simulink library "Renault_Library"  (Simulink 8.2)
settings: Solver=ode3  StartTime=0.0  StopTime=10.0  FixedStep=0.020  RelTol=1e-3
undefined symbols (not defined in the file or in its folder): ABC, AssystemProposal, b, chkval, CoeffA_C, CoeffB_C, CoeffMean_C, ConfTime_C, DiagDelay_C, DividePercent, FailureConfActive_C, FallingEdgeDuration_C, HighLevelThresholdVoltage_C, HighLevelVoltage_C, HighLevelVoltageTunable_C, InitValue_C, InputVoltRangeMax_C, InputVoltRangeMin_C, LowActiveContact_C, LowLevelThresholdVoltage_C, LowLevelVoltage_C, MaxOperatingVoltage_C, MinOperatingVoltage_C, mV2V_conversion, OUT1, OutOfRangeHigh_C, OutOfRangeLow_C, PinSupplySaturationMax, PinSupplySaturationMin, Res1Value_C, Res2Value_C, Res3Value_C, RisingEdgeDuration_C, RSA_CANSignalFailMonitoring, SampleTime_C, service14Table, service19Table, service22Table, service24Table, service28Table, service2ETable, service2FTable, service31Table, service87Table, SizeXStateDID2FTable, SizeXTable14, SizeXTable19, SizeXTable22, SizeXTable24, SizeXTable28, SizeXTable2E, SizeXTable2F, SizeXTable31, SizeXTable87, SizeYStateDID2FTable, SizeYTable14, SizeYTable19, SizeYTable22, SizeYTable24, SizeYTable28 ... (+22)
undefined symbols (not defined in the file): BatteryVoltage_ActivationThreshold_C, FrameStatus_AbsentValue_C, FrameStatus_ConfAbsentValue_C, MemThreshold_C, NAMEOFTHEMODEL_SAMPLE_TIME_CST, NbAbs1_C, NbConfAbs1_C, NbConfPres1_C, NbPres1_C, NOMDUSIGNAL_FaultConfTime_C, NOMDUSIGNAL_FaultRehabTime_C, NOMDUSIGNAL_switchvalue_C, NOMDUSIGNAL_unavailablevalue_C, PeriodFrame1_C, ResetTime_C, WakeUpTime_C
libraries: Renault_Library x112, simulink x75
(part 18 of 32)

== system Renault_Library/Services ECU/Diag/RSA_DiagnosticManagement/DIAGNOSTIC_SANCTION_TOP/Service11/SubFunction_In_Active_Session_Service11 ==
  DIAG_BUFFER_REQUEST : Inport
  SESSION : Inport  Port=2
  BitwiseOperator1 : simulink/Logic and Bit Operations/Bitwise Operator  logicop=AND  UseBitMask=on  BitMask=bin2dec('01111111')  BitMaskRealWorld=Stored Integer
  Constant{,11,13,15,18..20,22,24,27..28,35,37,39,42..43,49,51,53,56} (20 alike) : Constant  Value=true  OutDataTypeStr=boolean  SampleTime=-1
  Constant1 : Constant  Value=TYP_SESSION_ACTIVE.PROGRAMMING_SESSION  OutDataTypeStr=Inherit: Inherit via back propagation  SampleTime=-1
  Constant10 : Constant  Value=EnableRapidPowerShutDownSession1  OutDataTypeStr=Inherit: Inherit via back propagation  SampleTime=-1
  Constant12 : Constant  Value=HardResetSession1  OutDataTypeStr=Inherit: Inherit via back propagation  SampleTime=-1
  Constant14 : Constant  Value=KeyOffResetSession1  OutDataTypeStr=Inherit: Inherit via back propagation  SampleTime=-1
  Constant16 : Constant  Value=SoftResetSession1  OutDataTypeStr=Inherit: Inherit via back propagation  SampleTime=-1
  Constant17 : Constant  Value=DisableRapidPowerShutDownSession1  OutDataTypeStr=Inherit: Inherit via back propagation  SampleTime=-1
  Constant2 : Constant  Value=TYP_SESSION_ACTIVE.EXTENDED_SESSION  OutDataTypeStr=Inherit: Inherit via back propagation  SampleTime=-1
  Constant21 : Constant  Value=HardResetSession2  OutDataTypeStr=Inherit: Inherit via back propagation  SampleTime=-1
  Constant23 : Constant  Value=KeyOffResetSession2  OutDataTypeStr=Inherit: Inherit via back propagation  SampleTime=-1
  Constant25 : Constant  Value=SoftResetSession2  OutDataTypeStr=Inherit: Inherit via back propagation  SampleTime=-1
  Constant26 : Constant  Value=DisableRapidPowerShutDownSession2  OutDataTypeStr=Inherit: Inherit via back propagation  SampleTime=-1
  Constant{4,29,44,58} (4 alike) : Constant  Value=4  OutDataTypeStr=Inherit: Inherit via back propagation  SampleTime=-1
  Constant3 : Constant  Value=TYP_SESSION_ACTIVE.SAFETY_SYSTEM_SESSION  OutDataTypeStr=Inherit: Inherit via back propagation  SampleTime=-1
  Constant{5,30,45,59} (4 alike) : Constant  Value=5  OutDataTypeStr=Inherit: Inherit via back propagation  SampleTime=-1
  Constant{6,31,46,60} (4 alike) : Constant  Value=3  OutDataTypeStr=Inherit: Inherit via back propagation  SampleTime=-1
  Constant{32,47,61,65} (4 alike) : Constant  Value=2  OutDataTypeStr=Inherit: Inherit via back propagation  SampleTime=-1
  Constant{7,33,48,62} (4 alike) : Constant  OutDataTypeStr=Inherit: Inherit via back propagation  SampleTime=-1
  Constant34 : Constant  Value=EnableRapidPowerShutDownSession2  OutDataTypeStr=Inherit: Inherit via back propagation  SampleTime=-1
  Constant36 : Constant  Value=HardResetSession3  OutDataTypeStr=Inherit: Inherit via back propagation  SampleTime=-1
  Constant38 : Constant  Value=KeyOffResetSession3  OutDataTypeStr=Inherit: Inherit via back propagation  SampleTime=-1
  Constant40 : Constant  Value=SoftResetSession3  OutDataTypeStr=Inherit: Inherit via back propagation  SampleTime=-1
  Constant41 : Constant  Value=DisableRapidPowerShutDownSession3  OutDataTypeStr=Inherit: Inherit via back propagation  SampleTime=-1
  Constant50 : Constant  Value=HardResetSession4  OutDataTypeStr=Inherit: Inherit via back propagation  SampleTime=-1
  Constant52 : Constant  Value=KeyOffResetSession4  OutDataTypeStr=Inherit: Inherit via back propagation  SampleTime=-1
  Constant54 : Constant  Value=SoftResetSession4  OutDataTypeStr=Inherit: Inherit via back propagation  SampleTime=-1
  Constant55 : Constant  Value=DisableRapidPowerShutDownSession4  OutDataTypeStr=Inherit: Inherit via back propagation  SampleTime=-1
  Constant63 : Constant  Value=EnableRapidPowerShutDownSession4  OutDataTypeStr=Inherit: Inherit via back propagation  SampleTime=-1
  Constant8 : Constant  Value=TYP_SESSION_ACTIVE.DEFAULT_SESSION  OutDataTypeStr=Inherit: Inherit via back propagation  SampleTime=-1
  Constant9 : Constant  Value=EnableRapidPowerShutDownSession3  OutDataTypeStr=Inherit: Inherit via back propagation  SampleTime=-1
  LogicalOperator{,1..3,5..9,11..15,17..21,23,25..44} (40 alike) : Logic  OutDataTypeStr=boolean
  LogicalOperator{4,10,16,22} (4 alike) : Logic  Operator=OR  Inputs=5  OutDataTypeStr=boolean
  LogicalOperator24 : Logic  Operator=OR  Inputs=4  OutDataTypeStr=boolean
  RelationalOperator{1..43,45} (44 alike) : RelationalOperator  Operator===  OutDataTypeStr=boolean  InputSameDT=on
  Selector : Selector  InputPortWidth=4095  IndexOptions=Index vector (dialog)  Indices=[2]  OutputSizes=1
  SUBFUNCTION_SUPPORTED_IN_ACTIVE_SESSION : Outport
  wires:
  [Number_Subfunction] -> RelationalOperator40/1
  [Number_Subfunction] -> RelationalOperator38/1
  [Number_Subfunction] -> RelationalOperator41/1
  [Number_Subfunction] -> RelationalOperator39/1
  [Number_Subfunction] -> RelationalOperator42/1
  [Number_Subfunction] -> RelationalOperator31/1
  [Number_Subfunction] -> RelationalOperator29/1
  [Number_Subfunction] -> RelationalOperator32/1
  [Number_Subfunction] -> RelationalOperator30/1
  [Number_Subfunction] -> RelationalOperator33/1
  [Number_Subfunction] -> RelationalOperator21/1
  [Number_Subfunction] -> RelationalOperator19/1
  [Number_Subfunction] -> RelationalOperator22/1
  [Number_Subfunction] -> RelationalOperator20/1
  [Number_Subfunction] -> RelationalOperator23/1
  [Number_Subfunction] -> RelationalOperator5/1
  [Number_Subfunction] -> RelationalOperator4/1
  [Number_Subfunction] -> RelationalOperator6/1
  [Number_Subfunction] -> RelationalOperator45/1
  [Number_Subfunction] -> RelationalOperator7/1
  BitwiseOperator1/1 -> [Number_Subfunction]
  LogicalOperator24/1 -> SUBFUNCTION_SUPPORTED_IN_ACTIVE_SESSION/1
  LogicalOperator4/1 -> LogicalOperator24/1
  LogicalOperator22/1 -> LogicalOperator24/4
  LogicalOperator16/1 -> LogicalOperator24/3
  LogicalOperator10/1 -> LogicalOperator24/2
  RelationalOperator22/1 -> LogicalOperator32/1
  RelationalOperator19/1 -> LogicalOperator33/1
  RelationalOperator23/1 -> LogicalOperator30/1
  LogicalOperator32/1 -> LogicalOperator8/2
  Constant31/1 -> RelationalOperator22/2
  LogicalOperator33/1 -> LogicalOperator9/2
  LogicalOperator34/1 -> LogicalOperator11/2
  RelationalOperator21/1 -> LogicalOperator34/1
  Constant30/1 -> RelationalOperator21/2
  Constant29/1 -> RelationalOperator19/2
  RelationalOperator20/1 -> LogicalOperator31/1
  Constant32/1 -> RelationalOperator20/2
  RelationalOperator1/1 -> LogicalOperator30/2, LogicalOperator31/2, LogicalOperator32/2, LogicalOperator33/2, LogicalOperator34/2
  LogicalOperator30/1 -> LogicalOperator5/2
  LogicalOperator31/1 -> LogicalOperator7/2
  LogicalOperator11/1 -> LogicalOperator10/5
  RelationalOperator17/1 -> LogicalOperator11/1
  Constant27/1 -> RelationalOperator17/2
  Constant26/1 -> RelationalOperator17/1
  LogicalOperator9/1 -> LogicalOperator10/4
  LogicalOperator5/1 -> LogicalOperator10/1
  LogicalOperator8/1 -> LogicalOperator10/3
  LogicalOperator7/1 -> LogicalOperator10/2
  RelationalOperator18/1 -> LogicalOperator9/1
  Constant24/1 -> RelationalOperator18/2
  Constant34/1 -> RelationalOperator18/1
  RelationalOperator16/1 -> LogicalOperator8/1
  Constant25/1 -> RelationalOperator16/1
  Constant22/1 -> RelationalOperator16/2
  RelationalOperator15/1 -> LogicalOperator7/1
  Constant23/1 -> RelationalOperator15/1
  Constant20/1 -> RelationalOperator15/2
  RelationalOperator24/1 -> LogicalOperator5/1
  Constant19/1 -> RelationalOperator24/2
  Constant21/1 -> RelationalOperator24/1
  Constant33/1 -> RelationalOperator23/2
  RelationalOperator41/1 -> LogicalOperator42/1
  RelationalOperator38/1 -> LogicalOperator43/1
  RelationalOperator42/1 -> LogicalOperator40/1
  LogicalOperator42/1 -> LogicalOperator20/2
  Constant60/1 -> RelationalOperator41/2
  LogicalOperator43/1 -> LogicalOperator21/2
  LogicalOperator44/1 -> LogicalOperator23/2
  RelationalOperator40/1 -> LogicalOperator44/1
  Constant59/1 -> RelationalOperator40/2
  Constant58/1 -> RelationalOperator38/2
  RelationalOperator39/1 -> LogicalOperator41/1
  Constant61/1 -> RelationalOperator39/2
  RelationalOperator3/1 -> LogicalOperator40/2, LogicalOperator41/2, LogicalOperator42/2, LogicalOperator43/2, LogicalOperator44/2
  LogicalOperator40/1 -> LogicalOperator18/2
  LogicalOperator41/1 -> LogicalOperator19/2
  LogicalOperator23/1 -> LogicalOperator22/5
  RelationalOperator36/1 -> LogicalOperator23/1
  Constant56/1 -> RelationalOperator36/2
  Constant55/1 -> RelationalOperator36/1
  LogicalOperator21/1 -> LogicalOperator22/4
  LogicalOperator18/1 -> LogicalOperator22/1
  LogicalOperator20/1 -> LogicalOperator22/3
  LogicalOperator19/1 -> LogicalOperator22/2
  RelationalOperator37/1 -> LogicalOperator21/1
  Constant53/1 -> RelationalOperator37/2
  Constant63/1 -> RelationalOperator37/1
  RelationalOperator35/1 -> LogicalOperator20/1
  Constant54/1 -> RelationalOperator35/1
  Constant51/1 -> RelationalOperator35/2
  RelationalOperator34/1 -> LogicalOperator19/1
  Constant52/1 -> RelationalOperator34/1
  Constant49/1 -> RelationalOperator34/2
  RelationalOperator43/1 -> LogicalOperator18/1
  Constant43/1 -> RelationalOperator43/2
  Constant50/1 -> RelationalOperator43/1
  Constant62/1 -> RelationalOperator42/2
  RelationalOperator32/1 -> LogicalOperator37/1
  RelationalOperator29/1 -> LogicalOperator38/1
  RelationalOperator33/1 -> LogicalOperator35/1
  LogicalOperator37/1 -> LogicalOperator14/2
  Constant46/1 -> RelationalOperator32/2
  LogicalOperator38/1 -> LogicalOperator15/2
  LogicalOperator39/1 -> LogicalOperator17/2
  RelationalOperator31/1 -> LogicalOperator39/1
  Constant45/1 -> RelationalOperator31/2
  Constant44/1 -> RelationalOperator29/2
  RelationalOperator30/1 -> LogicalOperator36/1
  Constant47/1 -> RelationalOperator30/2
  RelationalOperator2/1 -> LogicalOperator35/2, LogicalOperator36/2, LogicalOperator37/2, LogicalOperator38/2, LogicalOperator39/2
  LogicalOperator35/1 -> LogicalOperator12/2
  LogicalOperator36/1 -> LogicalOperator13/2
  LogicalOperator17/1 -> LogicalOperator16/5
  RelationalOperator27/1 -> LogicalOperator17/1
  Constant42/1 -> RelationalOperator27/2
  Constant41/1 -> RelationalOperator27/1
  LogicalOperator15/1 -> LogicalOperator16/4
  LogicalOperator12/1 -> LogicalOperator16/1
  LogicalOperator14/1 -> LogicalOperator16/3
  LogicalOperator13/1 -> LogicalOperator16/2
  RelationalOperator28/1 -> LogicalOperator15/1
  Constant39/1 -> RelationalOperator28/2
  Constant9/1 -> RelationalOperator28/1
  RelationalOperator26/1 -> LogicalOperator14/1
  Constant40/1 -> RelationalOperator26/1
  Constant37/1 -> RelationalOperator26/2
  RelationalOperator25/1 -> LogicalOperator13/1
  Constant38/1 -> RelationalOperator25/1
  Constant35/1 -> RelationalOperator25/2
  RelationalOperator8/1 -> LogicalOperator12/1
  Constant28/1 -> RelationalOperator8/2
  Constant36/1 -> RelationalOperator8/1
  Constant48/1 -> RelationalOperator33/2
  RelationalOperator6/1 -> LogicalOperator27/1
  RelationalOperator4/1 -> LogicalOperator28/1
  RelationalOperator7/1 -> LogicalOperator25/1
  LogicalOperator27/1 -> LogicalOperator2/2
  Constant6/1 -> RelationalOperator6/2
  LogicalOperator28/1 -> LogicalOperator3/2
  LogicalOperator29/1 -> LogicalOperator6/2
  RelationalOperator5/1 -> LogicalOperator29/1
  Constant5/1 -> RelationalOperator5/2
  Constant4/1 -> RelationalOperator4/2
  RelationalOperator45/1 -> LogicalOperator26/1
  Constant65/1 -> RelationalOperator45/2
  RelationalOperator9/1 -> LogicalOperator25/2, LogicalOperator26/2, LogicalOperator27/2, LogicalOperator28/2, LogicalOperator29/2
  LogicalOperator25/1 -> LogicalOperator/2
  LogicalOperator26/1 -> LogicalOperator1/2
  LogicalOperator6/1 -> LogicalOperator4/5
  RelationalOperator13/1 -> LogicalOperator6/1
  Constant18/1 -> RelationalOperator13/2
  Constant17/1 -> RelationalOperator13/1
  LogicalOperator3/1 -> LogicalOperator4/4
  LogicalOperator/1 -> LogicalOperator4/1
  LogicalOperator2/1 -> LogicalOperator4/3
  LogicalOperator1/1 -> LogicalOperator4/2
  RelationalOperator14/1 -> LogicalOperator3/1
  Constant15/1 -> RelationalOperator14/2
  Constant10/1 -> RelationalOperator14/1
  RelationalOperator12/1 -> LogicalOperator2/1
  Constant16/1 -> RelationalOperator12/1
  Constant13/1 -> RelationalOperator12/2
  RelationalOperator11/1 -> LogicalOperator1/1
  Constant14/1 -> RelationalOperator11/1
  Constant11/1 -> RelationalOperator11/2
  RelationalOperator10/1 -> LogicalOperator/1
  Constant/1 -> RelationalOperator10/2
  Constant12/1 -> RelationalOperator10/1
  Constant7/1 -> RelationalOperator7/2
  DIAG_BUFFER_REQUEST/1 -> Selector/1
  Selector/1 -> BitwiseOperator1/1
  Constant3/1 -> RelationalOperator3/2
  Constant2/1 -> RelationalOperator2/2
  Constant1/1 -> RelationalOperator1/2
  SESSION/1 -> RelationalOperator3/1, RelationalOperator2/1, RelationalOperator1/1, RelationalOperator9/1
  Constant8/1 -> RelationalOperator9/2

== system Renault_Library/Services ECU/Diag/RSA_DiagnosticManagement/DIAGNOSTIC_SANCTION_TOP/Service11/SubFunction_Supported_Service11 ==
  DIAG_BUFFER_REQUEST : Inport
  BitwiseOperator1 : simulink/Logic and Bit Operations/Bitwise Operator  logicop=AND  UseBitMask=on  BitMask=bin2dec('01111111')  BitMaskRealWorld=Stored Integer
  BitwiseOperator4 : simulink/Logic and Bit Operations/Bitwise Operator  logicop=AND  UseBitMask=on  BitMask=bin2dec('10000000')  BitMaskRealWorld=Stored Integer
  Constant : Constant  OutDataTypeStr=Inherit: Inherit via back propagation  SampleTime=-1
  Constant1 : Constant  Value=2  OutDataTypeStr=Inherit: Inherit via back propagation  SampleTime=-1
  Constant10 : Constant  Value=EnableRapidPowerShutDown  OutDataTypeStr=Inherit: Inherit via back propagation  SampleTime=-1
  Constant{5,7,9,11..12} (5 alike) : Constant  Value=true  OutDataTypeStr=boolean  SampleTime=-1
  Constant16 : Constant  OutDataTypeStr=Inherit: Inherit via back propagation  SampleTime=-1
  Constant17 : Constant  Value=DisableRapidPowerShutDown  OutDataTypeStr=Inherit: Inherit via back propagation  SampleTime=-1
  Constant19 : Constant  Value=5  OutDataTypeStr=Inherit: Inherit via back propagation  SampleTime=-1
  Constant2 : Constant  Value=3  OutDataTypeStr=Inherit: Inherit via back propagation  SampleTime=-1
  Constant3 : Constant  Value=HardReset  OutDataTypeStr=Inherit: Inherit via back propagation  SampleTime=-1
  Constant4 : Constant  Value=4  OutDataTypeStr=Inherit: Inherit via back propagation  SampleTime=-1
  Constant6 : Constant  Value=KeyOffReset  OutDataTypeStr=Inherit: Inherit via back propagation  SampleTime=-1
  Constant8 : Constant  Value=SoftReset  OutDataTypeStr=Inherit: Inherit via back propagation  SampleTime=-1
  LogicalOperator{,1..3,6} (5 alike) : Logic  OutDataTypeStr=boolean
  LogicalOperator4 : Logic  Operator=OR  Inputs=5  OutDataTypeStr=boolean
  RelationalOperator{,1..7,12..13,16} (11 alike) : RelationalOperator  Operator===  OutDataTypeStr=boolean  InputSameDT=on
  Selector : Selector  InputPortWidth=4095  IndexOptions=Index vector (dialog)  Indices=[2]  OutputSizes=1
  ShiftArithmetic3 : ArithShift  BitShiftNumber=7
  SUPPRESS_POS_RES : Outport
  SUBFUNCTION_SUPPORTED : Outport  Port=2
  wires:
  LogicalOperator6/1 -> LogicalOperator4/5
  RelationalOperator12/1 -> LogicalOperator6/2
  RelationalOperator13/1 -> LogicalOperator6/1
  Constant12/1 -> RelationalOperator13/2
  Constant17/1 -> RelationalOperator13/1
  Constant19/1 -> RelationalOperator12/2
  BitwiseOperator1/1 -> RelationalOperator12/1, RelationalOperator3/1, RelationalOperator2/1, RelationalOperator1/1, RelationalOperator/1
  Constant16/1 -> RelationalOperator16/2
  Selector/1 -> BitwiseOperator1/1, BitwiseOperator4/1
  ShiftArithmetic3/1 -> RelationalOperator16/1
  BitwiseOperator4/1 -> ShiftArithmetic3/1
  RelationalOperator16/1 -> SUPPRESS_POS_RES/1
  RelationalOperator3/1 -> LogicalOperator3/2
  LogicalOperator4/1 -> SUBFUNCTION_SUPPORTED/1
  LogicalOperator3/1 -> LogicalOperator4/4
  LogicalOperator/1 -> LogicalOperator4/1
  LogicalOperator2/1 -> LogicalOperator4/3
  LogicalOperator1/1 -> LogicalOperator4/2
  RelationalOperator7/1 -> LogicalOperator3/1
  Constant11/1 -> RelationalOperator7/2
  Constant10/1 -> RelationalOperator7/1
  RelationalOperator6/1 -> LogicalOperator2/1
  RelationalOperator2/1 -> LogicalOperator2/2
  Constant8/1 -> RelationalOperator6/1
  Constant9/1 -> RelationalOperator6/2
  RelationalOperator5/1 -> LogicalOperator1/1
  RelationalOperator1/1 -> LogicalOperator1/2
  Constant6/1 -> RelationalOperator5/1
  Constant7/1 -> RelationalOperator5/2
  RelationalOperator4/1 -> LogicalOperator/1
  RelationalOperator/1 -> LogicalOperator/2
  Constant5/1 -> RelationalOperator4/2
  Constant3/1 -> RelationalOperator4/1
  Constant4/1 -> RelationalOperator3/2
  Constant2/1 -> RelationalOperator2/2
  Constant1/1 -> RelationalOperator1/2
  Constant/1 -> RelationalOperator/2
  DIAG_BUFFER_REQUEST/1 -> Selector/1

== system Renault_Library/Services ECU/Diag/RSA_DiagnosticManagement/DIAGNOSTIC_SANCTION_TOP/Service14/Check_Lenght_Message_Service14 ==
  REQ_DATA_NUMBER : Inport
  Constant1 : Constant  Value=4  OutDataTypeStr=Inherit: Inherit via back propagation  SampleTime=-1
  RelationalOperator1 : RelationalOperator  Operator===  OutDataTypeStr=boolean  InputSameDT=on
  LENGTH_MESSAGE_CORRECT : Outport
  wires:
  Constant1/1 -> RelationalOperator1/2
  REQ_DATA_NUMBER/1 -> RelationalOperator1/1
  RelationalOperator1/1 -> LENGTH_MESSAGE_CORRECT/1

== system Renault_Library/Services ECU/Diag/RSA_DiagnosticManagement/DIAGNOSTIC_SANCTION_TOP/Service14/Parameter_Valid_Service14 ==
  DIAG_BUFFER_REQUEST : Inport
  SESSION_ACTIVE : Inport  Port=2
  Parameter_service14 : Stateflow chart  TreatAsAtomicUnit=on
  PARAMETER_VALID : Outport
  wires:
  DIAG_BUFFER_REQUEST/1 -> Parameter_service14/1(DIAG_BUFFER_REQUEST)
  SESSION_ACTIVE/1 -> Parameter_service14/2(SESSION_ACTIVE)
  Parameter_service14/1(PARAMETER_VALID) -> PARAMETER_VALID/1

== system Renault_Library/Services ECU/Diag/RSA_DiagnosticManagement/DIAGNOSTIC_SANCTION_TOP/Service19/Check_Lenght_Message_Service19 ==
  REQ_DATA_NUMBER : Inport
  Constant1 : Constant  Value=2  OutDataTypeStr=Inherit: Inherit via back propagation  SampleTime=-1
  Constant8 : Constant  Value=4094  OutDataTypeStr=Inherit: Inherit via back propagation  SampleTime=-1
  LogicalOperator : Logic  OutDataTypeStr=boolean
  RelationalOperator1 : RelationalOperator  OutDataTypeStr=boolean  InputSameDT=on
  RelationalOperator9 : RelationalOperator  Operator=<  OutDataTypeStr=boolean  InputSameDT=on
  LENGTH_MESSAGE_CORRECT : Outport
  wires:
  REQ_DATA_NUMBER/1 -> RelationalOperator9/1, RelationalOperator1/1
  Constant8/1 -> RelationalOperator9/2
  Constant1/1 -> RelationalOperator1/2
  RelationalOperator9/1 -> LogicalOperator/1
  RelationalOperator1/1 -> LogicalOperator/2
  LogicalOperator/1 -> LENGTH_MESSAGE_CORRECT/1

== system Renault_Library/Services ECU/Diag/RSA_DiagnosticManagement/DIAGNOSTIC_SANCTION_TOP/Service19/Parameter_Valid_Service19 ==
  DIAG_BUFFER_REQUEST : Inport
  Parameter_service19 : Stateflow chart  TreatAsAtomicUnit=on
  PARAMETER_VALID : Outport
  wires:
  Parameter_service19/1(PARAMETER_VALID) -> PARAMETER_VALID/1
  DIAG_BUFFER_REQUEST/1 -> Parameter_service19/1(DIAG_BUFFER_REQUEST)

== system Renault_Library/Services ECU/Diag/RSA_DiagnosticManagement/DIAGNOSTIC_SANCTION_TOP/Service19/SubFunction_In_Active_Session_Service19 ==
  SESSION : Inport
  DIAG_BUFFER_REQUEST : Inport  Port=2
  BitwiseOperator1 : simulink/Logic and Bit Operations/Bitwise Operator  logicop=AND  UseBitMask=on  BitMask=bin2dec('01111111')  BitMaskRealWorld=Stored Integer
  Constant1 : Constant  Value=TYP_SESSION_ACTIVE.PROGRAMMING_SESSION  OutDataTypeStr=Inherit: Inherit via back propagation  SampleTime=-1
  Constant2 : Constant  Value=TYP_SESSION_ACTIVE.EXTENDED_SESSION  OutDataTypeStr=Inherit: Inherit via back propagation  SampleTime=-1
  Constant3 : Constant  Value=TYP_SESSION_ACTIVE.SAFETY_SYSTEM_SESSION  OutDataTypeStr=Inherit: Inherit via back propagation  SampleTime=-1
  Constant8 : Constant  Value=TYP_SESSION_ACTIVE.DEFAULT_SESSION  OutDataTypeStr=Inherit: Inherit via back propagation  SampleTime=-1
  LogicalOperator24 : Logic  Operator=OR  Inputs=4  OutDataTypeStr=boolean
  RelationalOperator{1..3,9} (4 alike) : RelationalOperator  Operator===  OutDataTypeStr=boolean  InputSameDT=on
  Selector : Selector  InputPortWidth=4095  IndexOptions=Index vector (dialog)  Indices=[2]  OutputSizes=1
  SubsystemDefaultSession_19 : Subsystem
  SubsystemExtenderdSession_19 : Subsystem
  SubsystemProgrammingSession_19 : Subsystem
  SubsystemSafetySession_19 : Subsystem
  SUBFUNCTION_SUPPORTED_IN_ACTIVE_SESSION : Outport
  wires:
  Constant8/1 -> RelationalOperator9/2
  SESSION/1 -> RelationalOperator9/1, RelationalOperator1/1, RelationalOperator2/1, RelationalOperator3/1
  Selector/1 -> BitwiseOperator1/1
  DIAG_BUFFER_REQUEST/1 -> Selector/1
  BitwiseOperator1/1 -> [Number_Subfunction]
  RelationalOperator9/1 -> SubsystemDefaultSession_19/1(Active_Session)
  LogicalOperator24/1 -> SUBFUNCTION_SUPPORTED_IN_ACTIVE_SESSION/1
  Constant1/1 -> RelationalOperator1/2
  RelationalOperator1/1 -> SubsystemProgrammingSession_19/1(Active_Session)
  Constant2/1 -> RelationalOperator2/2
  RelationalOperator2/1 -> SubsystemExtenderdSession_19/1(Active_Session)
  Constant3/1 -> RelationalOperator3/2
  RelationalOperator3/1 -> SubsystemSafetySession_19/1(Active_Session)
  SubsystemDefaultSession_19/1(Out1) -> LogicalOperator24/1
  SubsystemProgrammingSession_19/1(Out1) -> LogicalOperator24/2
  SubsystemExtenderdSession_19/1(Out1) -> LogicalOperator24/3
  SubsystemSafetySession_19/1(Out1) -> LogicalOperator24/4
  [Number_Subfunction] -> SubsystemDefaultSession_19/2(Number_Subfunction)
  [Number_Subfunction] -> SubsystemProgrammingSession_19/2(Number_Subfunction)
  [Number_Subfunction] -> SubsystemExtenderdSession_19/2(Number_Subfunction)
  [Number_Subfunction] -> SubsystemSafetySession_19/2(Number_Subfunction)

== system Renault_Library/Services ECU/Diag/RSA_DiagnosticManagement/DIAGNOSTIC_SANCTION_TOP/Service19/SubFunction_Supported_Service19 ==
  DIAG_BUFFER_REQUEST : Inport
  BitwiseOperator1 : simulink/Logic and Bit Operations/Bitwise Operator  logicop=AND  UseBitMask=on  BitMask=bin2dec('01111111')  BitMaskRealWorld=Stored Integer
  BitwiseOperator4 : simulink/Logic and Bit Operations/Bitwise Operator  logicop=AND  UseBitMask=on  BitMask=bin2dec('10000000')  BitMaskRealWorld=Stored Integer
  Constant : Constant  OutDataTypeStr=Inherit: Inherit via back propagation  SampleTime=-1
  Constant1 : Constant  Value=6  OutDataTypeStr=Inherit: Inherit via back propagation  SampleTime=-1
  Constant10 : Constant  Value=ReporDTCSnapshotIdentification  OutDataTypeStr=Inherit: Inherit via back propagation  SampleTime=-1
  Constant{5,9,11,13,18,20,23,28,30,34,36,40,56,58,60,62,65,69..74} (23 alike) : Constant  Value=true  OutDataTypeStr=boolean  SampleTime=-1
  Constant12 : Constant  Value=ReportDTCStoredDataByRecordNumber  OutDataTypeStr=Inherit: Inherit via back propagation  SampleTime=-1
  Constant14 : Constant  Value=5  OutDataTypeStr=Inherit: Inherit via back propagation  SampleTime=-1
  Constant15 : Constant  Value=ReportSupportedDTC  OutDataTypeStr=Inherit: Inherit via back propagation  SampleTime=-1
  Constant16 : Constant  OutDataTypeStr=Inherit: Inherit via back propagation  SampleTime=-1
  Constant17 : Constant  Value=ReporDTCSnapshotRecordByDTCNumber  OutDataTypeStr=Inherit: Inherit via back propagation  SampleTime=-1
  Constant19 : Constant  Value=4  OutDataTypeStr=Inherit: Inherit via back propagation  SampleTime=-1
  Constant2 : Constant  Value=2  OutDataTypeStr=Inherit: Inherit via back propagation  SampleTime=-1
  Constant21 : Constant  Value=10  OutDataTypeStr=Inherit: Inherit via back propagation  SampleTime=-1
  Constant22 : Constant  Value=ReportSeverityInformationOfDTC  OutDataTypeStr=Inherit: Inherit via back propagation  SampleTime=-1
  Constant24 : Constant  Value=9  OutDataTypeStr=Inherit: Inherit via back propagation  SampleTime=-1
  Constant25 : Constant  Value=7  OutDataTypeStr=Inherit: Inherit via back propagation  SampleTime=-1
  Constant26 : Constant  Value=ReportDTCExtendedDataRecordByDTCNumber  OutDataTypeStr=Inherit: Inherit via back propagation  SampleTime=-1
  Constant27 : Constant  Value=8  OutDataTypeStr=Inherit: Inherit via back propagation  SampleTime=-1
  Constant29 : Constant  Value=ReportNumberOfDTCBySeverityMaskRecord  OutDataTypeStr=Inherit: Inherit via back propagation  SampleTime=-1
  Constant3 : Constant  Value=ReportNumberOfDTCByStatusMask  OutDataTypeStr=Inherit: Inherit via back propagation  SampleTime=-1
  Constant31 : Constant  Value=11  OutDataTypeStr=Inherit: Inherit via back propagation  SampleTime=-1
  Constant32 : Constant  Value=16  OutDataTypeStr=Inherit: Inherit via back propagation  SampleTime=-1
  Constant33 : Constant  Value=ReportMostRecentTestFailedDTC  OutDataTypeStr=Inherit: Inherit via back propagation  SampleTime=-1
  Constant35 : Constant  Value=ReportMirrorMemoryDTCByStatusMask  OutDataTypeStr=Inherit: Inherit via back propagation  SampleTime=-1
  Constant37 : Constant  Value=15  OutDataTypeStr=Inherit: Inherit via back propagation  SampleTime=-1
  Constant38 : Constant  Value=ReportDTCFaultDetectionCounter  OutDataTypeStr=Inherit: Inherit via back propagation  SampleTime=-1
  Constant39 : Constant  Value=ReportMostRecentConfimedDTC  OutDataTypeStr=Inherit: Inherit via back propagation  SampleTime=-1
  Constant4 : Constant  Value=3  OutDataTypeStr=Inherit: Inherit via back propagation  SampleTime=-1
  Constant41 : Constant  Value=14  OutDataTypeStr=Inherit: Inherit via back propagation  SampleTime=-1
  Constant42 : Constant  Value=12  OutDataTypeStr=Inherit: Inherit via back propagation  SampleTime=-1
  Constant44 : Constant  Value=20  OutDataTypeStr=Inherit: Inherit via back propagation  SampleTime=-1
  Constant45 : Constant  Value=ReportEmissionsRelatedOBDDTCByStatusMask  OutDataTypeStr=Inherit: Inherit via back propagation  SampleTime=-1
  Constant47 : Constant  Value=19  OutDataTypeStr=Inherit: Inherit via back propagation  SampleTime=-1
  Constant48 : Constant  Value=17  OutDataTypeStr=Inherit: Inherit via back propagation  SampleTime=-1
  Constant49 : Constant  Value=ReportMirrorMemoryDTCExtendedDataRecordByDTCNumber  OutDataTypeStr=Inherit: Inherit via back propagation  SampleTime=-1
  Constant50 : Constant  Value=18  OutDataTypeStr=Inherit: Inherit via back propagation  SampleTime=-1
  Constant52 : Constant  Value=ReportNumberOfMirrorMemoryDTCByStatusMask  OutDataTypeStr=Inherit: Inherit via back propagation  SampleTime=-1
  Constant53 : Constant  Value=ReportFirstTestFailedDTC  OutDataTypeStr=Inherit: Inherit via back propagation  SampleTime=-1
  Constant55 : Constant  Value=13  OutDataTypeStr=Inherit: Inherit via back propagation  SampleTime=-1
  Constant57 : Constant  Value=ReportNumberOfEmissionsRelatedOBDDTCByStatusMask  OutDataTypeStr=Inherit: Inherit via back propagation  SampleTime=-1
  Constant59 : Constant  Value=ReportFirstConfirmedDTC  OutDataTypeStr=Inherit: Inherit via back propagation  SampleTime=-1
  Constant6 : Constant  Value=ReportDTCBySeverityMaskRecord  OutDataTypeStr=Inherit: Inherit via back propagation  SampleTime=-1
  Constant61 : Constant  Value=ReportWWHOBDDTCWithPermanentStatus  OutDataTypeStr=Inherit: Inherit via back propagation  SampleTime=-1
  Constant63 : Constant  Value=85  OutDataTypeStr=Inherit: Inherit via back propagation  SampleTime=-1
  Constant64 : Constant  Value=ReportWWHOBDDTCByMaskRecord  OutDataTypeStr=Inherit: Inherit via back propagation  SampleTime=-1
  Constant66 : Constant  Value=66  OutDataTypeStr=Inherit: Inherit via back propagation  SampleTime=-1
  Constant67 : Constant  Value=21  OutDataTypeStr=Inherit: Inherit via back propagation  SampleTime=-1
  Constant68 : Constant  Value=ReportDTCWithPermanetStatus  OutDataTypeStr=Inherit: Inherit via back propagation  SampleTime=-1
  Constant8 : Constant  Value=ReportDTCByStatusMask  OutDataTypeStr=Inherit: Inherit via back propagation  SampleTime=-1
  LogicalOperator{,1..3,5..23} (23 alike) : Logic  OutDataTypeStr=boolean
  LogicalOperator4 : Logic  Operator=OR  Inputs=23  OutDataTypeStr=boolean
  RelationalOperator{,1..46} (47 alike) : RelationalOperator  Operator===  OutDataTypeStr=boolean  InputSameDT=on
  Selector : Selector  InputPortWidth=4095  IndexOptions=Index vector (dialog)  Indices=[2]  OutputSizes=1
  ShiftArithmetic3 : ArithShift  BitShiftNumber=7
  SUBFUNCTION_SUPPORTED : Outport
  SUPPRESS_POS_RES : Outport  Port=2
  wires:
  DIAG_BUFFER_REQUEST/1 -> Selector/1
  Constant/1 -> RelationalOperator/2
  Constant2/1 -> RelationalOperator2/2
  Constant4/1 -> RelationalOperator3/2
  Constant3/1 -> RelationalOperator4/1
  Constant5/1 -> RelationalOperator4/2
  RelationalOperator/1 -> LogicalOperator/2
  RelationalOperator4/1 -> LogicalOperator/1
  Constant36/1 -> RelationalOperator6/2
  Constant8/1 -> RelationalOperator6/1
  RelationalOperator2/1 -> LogicalOperator2/2
  RelationalOperator6/1 -> LogicalOperator2/1
  Constant10/1 -> RelationalOperator7/1
  Constant56/1 -> RelationalOperator7/2
  RelationalOperator7/1 -> LogicalOperator3/1
  RelationalOperator3/1 -> LogicalOperator3/2
  RelationalOperator16/1 -> SUPPRESS_POS_RES/1
  BitwiseOperator4/1 -> ShiftArithmetic3/1
  ShiftArithmetic3/1 -> RelationalOperator16/1
  Selector/1 -> BitwiseOperator4/1, BitwiseOperator1/1
  Constant16/1 -> RelationalOperator16/2
  BitwiseOperator1/1 -> RelationalOperator/1, RelationalOperator2/1, RelationalOperator3/1, RelationalOperator12/1, RelationalOperator8/1, RelationalOperator1/1, RelationalOperator11/1, RelationalOperator14/1, RelationalOperator5/1, RelationalOperator19/1, RelationalOperator21/1, RelationalOperator32/1, RelationalOperator34/1, RelationalOperator25/1, RelationalOperator39/1, RelationalOperator22/1, RelationalOperator24/1, RelationalOperator27/1, RelationalOperator36/1, RelationalOperator31/1, RelationalOperator42/1, RelationalOperator46/1, RelationalOperator44/1
  Constant19/1 -> RelationalOperator12/2
  Constant17/1 -> RelationalOperator13/1
  Constant58/1 -> RelationalOperator13/2
  RelationalOperator13/1 -> LogicalOperator6/1
  RelationalOperator12/1 -> LogicalOperator6/2
  Constant14/1 -> RelationalOperator8/2
  Constant12/1 -> RelationalOperator9/1
  Constant71/1 -> RelationalOperator9/2
  RelationalOperator9/1 -> LogicalOperator5/1
  RelationalOperator8/1 -> LogicalOperator5/2
  Constant1/1 -> RelationalOperator1/2
  Constant25/1 -> RelationalOperator11/2
  Constant27/1 -> RelationalOperator14/2
  Constant60/1 -> RelationalOperator15/2
  RelationalOperator1/1 -> LogicalOperator1/2
  RelationalOperator15/1 -> LogicalOperator1/1
  Constant62/1 -> RelationalOperator17/2
  Constant29/1 -> RelationalOperator17/1
  RelationalOperator11/1 -> LogicalOperator7/2
  RelationalOperator17/1 -> LogicalOperator7/1
  Constant6/1 -> RelationalOperator18/1
  Constant65/1 -> RelationalOperator18/2
  RelationalOperator18/1 -> LogicalOperator8/1
  RelationalOperator14/1 -> LogicalOperator8/2
  Constant24/1 -> RelationalOperator5/2
  Constant22/1 -> RelationalOperator10/1
  Constant69/1 -> RelationalOperator10/2
  RelationalOperator10/1 -> LogicalOperator10/1
  RelationalOperator5/1 -> LogicalOperator10/2
  Constant21/1 -> RelationalOperator19/2
  Constant15/1 -> RelationalOperator20/1
  Constant70/1 -> RelationalOperator20/2
  RelationalOperator20/1 -> LogicalOperator9/1
  RelationalOperator19/1 -> LogicalOperator9/2
  Constant31/1 -> RelationalOperator21/2
  Constant42/1 -> RelationalOperator32/2
  Constant55/1 -> RelationalOperator34/2
  Constant53/1 -> RelationalOperator35/1
  Constant72/1 -> RelationalOperator35/2
  RelationalOperator21/1 -> LogicalOperator11/2
  RelationalOperator35/1 -> LogicalOperator11/1
  Constant73/1 -> RelationalOperator37/2
  Constant59/1 -> RelationalOperator37/1
  RelationalOperator32/1 -> LogicalOperator14/2
  RelationalOperator37/1 -> LogicalOperator14/1
  Constant33/1 -> RelationalOperator38/1
  Constant11/1 -> RelationalOperator38/2
  RelationalOperator38/1 -> LogicalOperator15/1
  RelationalOperator34/1 -> LogicalOperator15/2
  Constant41/1 -> RelationalOperator25/2
  Constant39/1 -> RelationalOperator26/1
  Constant9/1 -> RelationalOperator26/2
  RelationalOperator26/1 -> LogicalOperator17/1
  RelationalOperator25/1 -> LogicalOperator17/2
  Constant37/1 -> RelationalOperator39/2
  Constant35/1 -> RelationalOperator40/1
  Constant13/1 -> RelationalOperator40/2
  RelationalOperator40/1 -> LogicalOperator16/1
  RelationalOperator39/1 -> LogicalOperator16/2
  Constant32/1 -> RelationalOperator22/2
  Constant48/1 -> RelationalOperator24/2
  Constant50/1 -> RelationalOperator27/2
  Constant18/1 -> RelationalOperator28/2
  RelationalOperator22/1 -> LogicalOperator12/2
  RelationalOperator28/1 -> LogicalOperator12/1
  Constant20/1 -> RelationalOperator29/2
  Constant52/1 -> RelationalOperator29/1
  RelationalOperator24/1 -> LogicalOperator18/2
  RelationalOperator29/1 -> LogicalOperator18/1
  Constant57/1 -> RelationalOperator30/1
  Constant23/1 -> RelationalOperator30/2
  RelationalOperator30/1 -> LogicalOperator19/1
  RelationalOperator27/1 -> LogicalOperator19/2
  Constant47/1 -> RelationalOperator36/2
  Constant45/1 -> RelationalOperator23/1
  Constant74/1 -> RelationalOperator23/2
  RelationalOperator23/1 -> LogicalOperator13/1
  RelationalOperator36/1 -> LogicalOperator13/2
  Constant38/1 -> RelationalOperator33/1
  Constant28/1 -> RelationalOperator33/2
  RelationalOperator33/1 -> LogicalOperator20/1
  RelationalOperator31/1 -> LogicalOperator20/2
  Constant44/1 -> RelationalOperator31/2
  Constant67/1 -> RelationalOperator42/2
  Constant68/1 -> RelationalOperator43/1
  Constant30/1 -> RelationalOperator43/2
  RelationalOperator43/1 -> LogicalOperator22/1
  RelationalOperator42/1 -> LogicalOperator22/2
  Constant66/1 -> RelationalOperator46/2
  Constant64/1 -> RelationalOperator41/1
  Constant34/1 -> RelationalOperator41/2
  RelationalOperator41/1 -> LogicalOperator21/1
  RelationalOperator46/1 -> LogicalOperator21/2
  Constant61/1 -> RelationalOperator45/1
  Constant40/1 -> RelationalOperator45/2
  RelationalOperator45/1 -> LogicalOperator23/1
  RelationalOperator44/1 -> LogicalOperator23/2
  Constant63/1 -> RelationalOperator44/2
  LogicalOperator/1 -> LogicalOperator4/1
  LogicalOperator2/1 -> LogicalOperator4/2
  LogicalOperator3/1 -> LogicalOperator4/3
  LogicalOperator6/1 -> LogicalOperator4/4
  LogicalOperator5/1 -> LogicalOperator4/5
  LogicalOperator1/1 -> LogicalOperator4/6
  LogicalOperator7/1 -> LogicalOperator4/7
  LogicalOperator8/1 -> LogicalOperator4/8
  LogicalOperator10/1 -> LogicalOperator4/9
  LogicalOperator9/1 -> LogicalOperator4/10
  LogicalOperator11/1 -> LogicalOperator4/11
  LogicalOperator14/1 -> LogicalOperator4/12
  LogicalOperator15/1 -> LogicalOperator4/13
  LogicalOperator17/1 -> LogicalOperator4/14
  LogicalOperator16/1 -> LogicalOperator4/15
  LogicalOperator12/1 -> LogicalOperator4/16
  LogicalOperator18/1 -> LogicalOperator4/17
  LogicalOperator19/1 -> LogicalOperator4/18
  LogicalOperator13/1 -> LogicalOperator4/19
  LogicalOperator20/1 -> LogicalOperator4/20
  LogicalOperator22/1 -> LogicalOperator4/21
  LogicalOperator21/1 -> LogicalOperator4/22
  LogicalOperator23/1 -> LogicalOperator4/23
  Constant26/1 -> RelationalOperator15/1
  Constant49/1 -> RelationalOperator28/1
  LogicalOperator4/1 -> SUBFUNCTION_SUPPORTED/1

== system Renault_Library/Services ECU/Diag/RSA_DiagnosticManagement/DIAGNOSTIC_SANCTION_TOP/Service22/Check_Lenght_Message_Response_Service22 ==
  RESPONSE_SIZE : Inport
  Constant8 : Constant  Value=4094  OutDataTypeStr=Inherit: Inherit via back propagation  SampleTime=-1
  RelationalOperator9 : RelationalOperator  Operator=<  OutDataTypeStr=boolean  InputSameDT=on
  LENGTH_RESPONSE_CORRECT : Outport
  wires:
  RESPONSE_SIZE/1 -> RelationalOperator9/1
  Constant8/1 -> RelationalOperator9/2
  RelationalOperator9/1 -> LENGTH_RESPONSE_CORRECT/1

== system Renault_Library/Services ECU/Diag/RSA_DiagnosticManagement/DIAGNOSTIC_SANCTION_TOP/Service22/Check_Lenght_Message_Service22 ==
  REQ_DATA_NUMBER : Inport
  Constant1 : Constant  Value=3  OutDataTypeStr=Inherit: Inherit via back propagation  SampleTime=-1
  Constant8 : Constant  Value=4094  OutDataTypeStr=Inherit: Inherit via back propagation  SampleTime=-1
  LogicalOperator : Logic  OutDataTypeStr=boolean
  RelationalOperator1 : RelationalOperator  OutDataTypeStr=boolean  InputSameDT=on
  RelationalOperator9 : RelationalOperator  Operator=<  OutDataTypeStr=boolean  InputSameDT=on
  LENGTH_MESSAGE_CORRECT : Outport
  wires:
  REQ_DATA_NUMBER/1 -> RelationalOperator9/1, RelationalOperator1/1
  Constant8/1 -> RelationalOperator9/2
  Constant1/1 -> RelationalOperator1/2
  RelationalOperator9/1 -> LogicalOperator/1
  RelationalOperator1/1 -> LogicalOperator/2
  LogicalOperator/1 -> LENGTH_MESSAGE_CORRECT/1

== system Renault_Library/Services ECU/Diag/RSA_DiagnosticManagement/DIAGNOSTIC_SANCTION_TOP/Service22/Parameter_Valid_Service22 ==
  REQ_DATA_NUMBER : Inport
  DIAG_BUFFER_REQUEST : Inport  Port=2
  SESSION_ACTIVE : Inport  Port=3
  Parameter_service22 : Stateflow chart  TreatAsAtomicUnit=on
  RESPONSE_SIZE : Outport
  SECURITY_ACCESS_ACCEPTED : Outport  Port=2
  PARAMETER_VALID : Outport  Port=3
  LIST_DID_SERVICE_22 : Outport  Port=4  PortDimensions=[2046 3]
  wires:
  DIAG_BUFFER_REQUEST/1 -> Parameter_service22/1(DIAG_BUFFER_REQUEST)
  REQ_DATA_NUMBER/1 -> Parameter_service22/2(REQ_DATA_NUMBER)
  Parameter_service22/1(PARAMETER_VALID) -> PARAMETER_VALID/1
  Parameter_service22/2(LIST_DID_SERVICE_22) -> LIST_DID_SERVICE_22/1
  Parameter_service22/3(RESPONSE_SIZE) -> RESPONSE_SIZE/1
  Parameter_service22/4(SECURITY_ACCESS_ACCEPTED) -> SECURITY_ACCESS_ACCEPTED/1
  SESSION_ACTIVE/1 -> Parameter_service22/3(SESSION_ACTIVE)

== system Renault_Library/Services ECU/Diag/RSA_DiagnosticManagement/DIAGNOSTIC_SANCTION_TOP/Service23/Check_Lenght_Message_Service23 ==
  REQ_DATA_NUMBER : Inport
  Constant1 : Constant  Value=4  OutDataTypeStr=Inherit: Inherit via back propagation  SampleTime=-1
  Constant8 : Constant  Value=4094  OutDataTypeStr=Inherit: Inherit via back propagation  SampleTime=-1
  LogicalOperator : Logic  OutDataTypeStr=boolean
  RelationalOperator1 : RelationalOperator  OutDataTypeStr=boolean  InputSameDT=on
  RelationalOperator9 : RelationalOperator  Operator=<  OutDataTypeStr=boolean  InputSameDT=on
  LENGTH_MESSAGE_CORRECT : Outport
  wires:
  REQ_DATA_NUMBER/1 -> RelationalOperator9/1, RelationalOperator1/1
  Constant8/1 -> RelationalOperator9/2
  Constant1/1 -> RelationalOperator1/2
  RelationalOperator9/1 -> LogicalOperator/1
  RelationalOperator1/1 -> LogicalOperator/2
  LogicalOperator/1 -> LENGTH_MESSAGE_CORRECT/1

== system Renault_Library/Services ECU/Diag/RSA_DiagnosticManagement/DIAGNOSTIC_SANCTION_TOP/Service23/Parameter_Valid_Service23 ==
  REQ_DATA_NUMBER : Inport
  DIAG_BUFFER_REQUEST : Inport  Port=2
  SESSION_ACTIVE : Inport  Port=3
  Parameter_service23 : Stateflow chart  TreatAsAtomicUnit=on
  SECURITY_ACCESS_ACCEPTED : Outport
  PARAMETER_VALID : Outport  Port=2
  wires:
  DIAG_BUFFER_REQUEST/1 -> Parameter_service23/1(DIAG_BUFFER_REQUEST)
  REQ_DATA_NUMBER/1 -> Parameter_service23/2(REQ_DATA_NUMBER)
  Parameter_service23/1(PARAMETER_VALID) -> PARAMETER_VALID/1
  Parameter_service23/2(SECURITY_ACCESS_ACCEPTED) -> SECURITY_ACCESS_ACCEPTED/1
  SESSION_ACTIVE/1 -> Parameter_service23/3(SESSION_ACTIVE)

== system Renault_Library/Services ECU/Diag/RSA_DiagnosticManagement/DIAGNOSTIC_SANCTION_TOP/Service24/Check_Lenght_Message_Service24 ==
  REQ_DATA_NUMBER : Inport
  Constant8 : Constant  Value=3  OutDataTypeStr=Inherit: Inherit via back propagation  SampleTime=-1
  RelationalOperator9 : RelationalOperator  Operator===  OutDataTypeStr=boolean  InputSameDT=on
  LENGTH_MESSAGE_CORRECT : Outport
  wires:
  REQ_DATA_NUMBER/1 -> RelationalOperator9/1
  Constant8/1 -> RelationalOperator9/2
  RelationalOperator9/1 -> LENGTH_MESSAGE_CORRECT/1

== system Renault_Library/Services ECU/Diag/RSA_DiagnosticManagement/DIAGNOSTIC_SANCTION_TOP/Service24/Parameter_Valid_Service24 ==
  DIAG_BUFFER_REQUEST : Inport
  SESSION_ACTIVE : Inport  Port=2
  Parameter_service24 : Stateflow chart  TreatAsAtomicUnit=on
  SECURITY_ACCESS_ACCEPTED : Outport
  PARAMETER_VALID : Outport  Port=2
  wires:
  DIAG_BUFFER_REQUEST/1 -> Parameter_service24/1(DIAG_BUFFER_REQUEST)
  Parameter_service24/1(PARAMETER_VALID) -> PARAMETER_VALID/1
  Parameter_service24/2(SECURITY_ACCESS_ACCEPTED) -> SECURITY_ACCESS_ACCEPTED/1
  SESSION_ACTIVE/1 -> Parameter_service24/2(SESSION_ACTIVE)

== system Renault_Library/Services ECU/Diag/RSA_DiagnosticManagement/DIAGNOSTIC_SANCTION_TOP/Service27/Check_Lenght_Message_Service27 ==
  REQ_DATA_NUMBER : Inport
  Constant1 : Constant  Value=2  OutDataTypeStr=Inherit: Inherit via back propagation  SampleTime=-1
  Constant8 : Constant  Value=4094  OutDataTypeStr=Inherit: Inherit via back propagation  SampleTime=-1
  LogicalOperator : Logic  OutDataTypeStr=boolean
  RelationalOperator1 : RelationalOperator  OutDataTypeStr=boolean  InputSameDT=on
  RelationalOperator9 : RelationalOperator  Operator=<  OutDataTypeStr=boolean  InputSameDT=on
  LENGTH_MESSAGE_CORRECT : Outport
  wires:
  REQ_DATA_NUMBER/1 -> RelationalOperator9/1, RelationalOperator1/1
  Constant8/1 -> RelationalOperator9/2
  Constant1/1 -> RelationalOperator1/2
  RelationalOperator9/1 -> LogicalOperator/1
  RelationalOperator1/1 -> LogicalOperator/2
  LogicalOperator/1 -> LENGTH_MESSAGE_CORRECT/1
